simulink model "fftwbr_core"  (Simulink 7.9)
settings: Solver=ode45  StartTime=0.0  StopTime=10.0  RelTol=1e-3
libraries: xbsIndex_r4 x15792, casper_library_misc x464, casper_library_delays x57, xbsReplacements_r4 x8, casper_library_ffts_twiddle x4
(part 123 of 233)

== system fftwbr_core/fft_wideband_real1/fft_direct/butterfly3_6/twiddle_general_dsp48e/coeff_gen ==
  rst : Inport
  Counter : xbsIndex_r4/Counter  cnt_type=Free Running  cnt_to=Inf  operation=Up  start_count=0  cnt_by_val=1  arith_type=Unsigned  n_bits=11  bin_pt=0  load_pin=off  rst=on  en=off  explicit_period=on  period=1  dbl_ovrd=off  use_behavioral_HDL=off  implementation=Fabric  block_type=counter
  ROM : xbsIndex_r4/ROM  depth=2048  initVector="[-0.7071075439453125                    -0.7073822021484375                       -0.7076416015625                     -0.707916259765625                      -0.70819091796875                     -0.708465576171875                    -0.7087249755859375                    -0.7089996337890625                    -0.7092742919921875                    -0.7095489501953125                     -0.709808349609375                       -0.7100830078125                     -0.710357666015625                    -0.7106170654296875                    -0.7108917236328125                    -0.7111663818359375                         -0.71142578125                     -0.711700439453125                      -0.71197509765625                    -0.7122344970703125                    -0.7125091552734375                    -0.7127838134765625                     -0.713043212890625                      -0.71331787109375                    -0.7135772705078125                    -0.7138519287109375                    -0.7141265869140625                     -0.714385986328125                      -0.71466064453125                    -0.7149200439453125                    -0.7151947021484375                    -0.7154693603515625                     -0.715728759765625                      -0.71600341796875                    -0.7162628173828125                    -0.7165374755859375                           -0.716796875                     -0.717071533203125                    -0.7173309326171875                    -0.7176055908203125                     -0.717864990234375                       -0.7181396484375                    -0.7183990478515625                    -0.7186737060546875                      -0.71893310546875                     -0.719207763671875                    -0.7194671630859375                    -0.7197418212890625                     -0.720001220703125                      -0.72027587890625                    -0.7205352783203125                     -0.720794677734375                       -0.7210693359375                    -0.7213287353515625                    -0.7216033935546875                      -0.72186279296875                    -0.7221221923828125                    -0.7223968505859375                            -0.72265625                     -0.722930908203125                    -0.7231903076171875                      -0.72344970703125                     -0.723724365234375                    -0.7239837646484375                       -0.7242431640625                     -0.724517822265625                    -0.7247772216796875                      -0.72503662109375                     -0.725311279296875                    -0.7255706787109375                        -0.725830078125                    -0.7260894775390625                    -0.7263641357421875                      -0.72662353515625                    -0.7268829345703125                     -0.727142333984375                       -0.7274169921875                    -0.7276763916015625                     -0.727935791015625                    -0.7281951904296875                    -0.7284698486328125                     -0.728729248046875                    -0.7289886474609375                        -0.729248046875                    -0.7295074462890625                    -0.7297821044921875                      -0.73004150390625                    -0.7303009033203125                     -0.730560302734375                    -0.7308197021484375                       -0.7310791015625                     -0.731353759765625                    -0.7316131591796875                      -0.73187255859375                    -0.7321319580078125                     -0.732391357421875                    -0.7326507568359375                         -0.73291015625                    -0.7331695556640625                    -0.7334442138671875                      -0.73370361328125                    -0.7339630126953125                     -0.734222412109375 ...(+75854 chars)]"  distributed_mem=Block RAM  rst=off  init_reg=0  en=off  latency=2  gui_display_data_type=Fixed-point  arith_type="Signed  (2's comp)"  n_bits=18  bin_pt=16  preci_type=Single  exp_width=8  frac_width=24  dbl_ovrd=off  optimize=Area  use_rpm=on  block_type=sprom
  ROM1 : xbsIndex_r4/ROM  depth=2048  initVector="[-0.7071075439453125                    -0.7068328857421875                    -0.7065582275390625                        -0.706298828125                     -0.706024169921875                      -0.70574951171875                     -0.705474853515625                       -0.7052001953125                    -0.7049407958984375                    -0.7046661376953125                    -0.7043914794921875                    -0.7041168212890625                    -0.7038421630859375                    -0.7035675048828125                    -0.7032928466796875                     -0.703033447265625                       -0.7027587890625                     -0.702484130859375                      -0.70220947265625                     -0.701934814453125                         -0.70166015625                     -0.701385498046875                      -0.70111083984375                     -0.700836181640625                       -0.7005615234375                    -0.7003021240234375                    -0.7000274658203125                    -0.6997528076171875                    -0.6994781494140625                    -0.6992034912109375                    -0.6989288330078125                    -0.6986541748046875                    -0.6983795166015625                    -0.6981048583984375                    -0.6978302001953125                    -0.6975555419921875                    -0.6972808837890625                    -0.6970062255859375                    -0.6967315673828125                    -0.6964569091796875                    -0.6961822509765625                    -0.6959075927734375                    -0.6956329345703125                    -0.6953582763671875                        -0.695068359375                     -0.694793701171875                      -0.69451904296875                     -0.694244384765625                       -0.6939697265625                     -0.693695068359375                      -0.69342041015625                     -0.693145751953125                         -0.69287109375                     -0.692596435546875                    -0.6923065185546875                    -0.6920318603515625                    -0.6917572021484375                    -0.6914825439453125                    -0.6912078857421875                    -0.6909332275390625                     -0.690643310546875                      -0.69036865234375                     -0.690093994140625                       -0.6898193359375                     -0.689544677734375                      -0.68927001953125                    -0.6889801025390625                    -0.6887054443359375                    -0.6884307861328125                    -0.6881561279296875                       -0.6878662109375                     -0.687591552734375                      -0.68731689453125                     -0.687042236328125                    -0.6867523193359375                    -0.6864776611328125                    -0.6862030029296875                    -0.6859283447265625                     -0.685638427734375                      -0.68536376953125                     -0.685089111328125                    -0.6847991943359375                    -0.6845245361328125                    -0.6842498779296875                       -0.6839599609375                     -0.683685302734375                      -0.68341064453125                    -0.6831207275390625                    -0.6828460693359375                    -0.6825714111328125                     -0.682281494140625                       -0.6820068359375                    -0.6817169189453125                    -0.6814422607421875                    -0.6811676025390625                     -0.680877685546875                      -0.68060302734375                    -0.6803131103515625                    -0.6800384521484375                    -0.6797637939453125                     -0.679473876953125                         -0.67919921875                    -0.6789093017578125 ...(+75854 chars)]"  distributed_mem=Block RAM  rst=off  init_reg=0  en=off  latency=2  gui_display_data_type=Fixed-point  arith_type="Signed  (2's comp)"  n_bits=18  bin_pt=16  preci_type=Single  exp_width=8  frac_width=24  dbl_ovrd=off  optimize=Area  use_rpm=on  block_type=sprom
  Slice : xbsIndex_r4/Slice  nbits=11  boolean_output=off  mode=Upper Bit Location + Width  bit1=0  base1=MSB of Input  bit0=0  base0=LSB of Input  dbl_ovrd=off  block_type=slice
  ri_to_c : casper_library_misc/ri_to_c
  w : Outport
  wires:
  ri_to_c/1 -> w/1
  rst/1 -> Counter/1
  Counter/1 -> Slice/1
  Slice/1 -> ROM/1, ROM1/1
  ROM/1 -> ri_to_c/1
  ROM1/1 -> ri_to_c/2
